simulink model "HvacCtrlrAirFlowDistbnFrnt"  (R2022b)
settings: Solver=FixedStepDiscrete  StartTime=0.0  StopTime=10.0  FixedStep=0.2  RelTol=1e-3
(part 2 of 2)

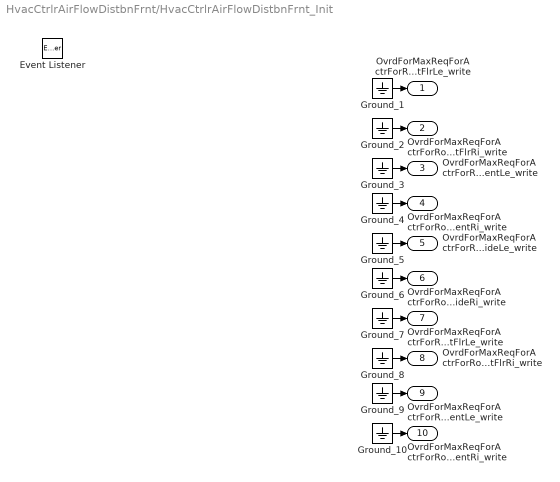
== system HvacCtrlrAirFlowDistbnFrnt/HvacCtrlrAirFlowDistbnFrnt_Init ==
  Event Listener : EventListener  EventName=reset
  Ground_{1..10} (10 alike) : Ground
  OvrdForMaxReqForActrForRowFirstAtFlr_OvrdForMaxReqForActrForRowFirstAtFlrLe_write : Outport
  OvrdForMaxReqForActrForRowFirstAtFlr_OvrdForMaxReqForActrForRowFirstAtFlrRi_write : Outport  Port=2
  OvrdForMaxReqForActrForRowFirstAtVent_OvrdForMaxReqForActrForRowFirstAtVentLe_write : Outport  Port=3
  OvrdForMaxReqForActrForRowFirstAtVent_OvrdForMaxReqForActrForRowFirstAtVentRi_write : Outport  Port=4
  OvrdForMaxReqForActrForRowFirstAtVent_OvrdForMaxReqForActrForRowFirstAtVentSideLe_write : Outport  Port=5
  OvrdForMaxReqForActrForRowFirstAtVent_OvrdForMaxReqForActrForRowFirstAtVentSideRi_write : Outport  Port=6
  OvrdForMaxReqForActrForRowSecAtFlr_OvrdForMaxReqForActrForRowSecAtFlrLe_write : Outport  Port=7
  OvrdForMaxReqForActrForRowSecAtFlr_OvrdForMaxReqForActrForRowSecAtFlrRi_write : Outport  Port=8
  OvrdForMaxReqForActrForRowSecAtVent_OvrdForMaxReqForActrForRowSecAtVentLe_write : Outport  Port=9
  OvrdForMaxReqForActrForRowSecAtVent_OvrdForMaxReqForActrForRowSecAtVentRi_write : Outport  Port=10
  wires:
  Ground_1/1 -> OvrdForMaxReqForActrForRowFirstAtFlr_OvrdForMaxReqForActrForRowFirstAtFlrLe_write/1
  Ground_2/1 -> OvrdForMaxReqForActrForRowFirstAtFlr_OvrdForMaxReqForActrForRowFirstAtFlrRi_write/1
  Ground_3/1 -> OvrdForMaxReqForActrForRowFirstAtVent_OvrdForMaxReqForActrForRowFirstAtVentLe_write/1
  Ground_4/1 -> OvrdForMaxReqForActrForRowFirstAtVent_OvrdForMaxReqForActrForRowFirstAtVentRi_write/1
  Ground_5/1 -> OvrdForMaxReqForActrForRowFirstAtVent_OvrdForMaxReqForActrForRowFirstAtVentSideLe_write/1
  Ground_6/1 -> OvrdForMaxReqForActrForRowFirstAtVent_OvrdForMaxReqForActrForRowFirstAtVentSideRi_write/1
  Ground_7/1 -> OvrdForMaxReqForActrForRowSecAtFlr_OvrdForMaxReqForActrForRowSecAtFlrLe_write/1
  Ground_8/1 -> OvrdForMaxReqForActrForRowSecAtFlr_OvrdForMaxReqForActrForRowSecAtFlrRi_write/1
  Ground_9/1 -> OvrdForMaxReqForActrForRowSecAtVent_OvrdForMaxReqForActrForRowSecAtVentLe_write/1
  Ground_10/1 -> OvrdForMaxReqForActrForRowSecAtVent_OvrdForMaxReqForActrForRowSecAtVentRi_write/1

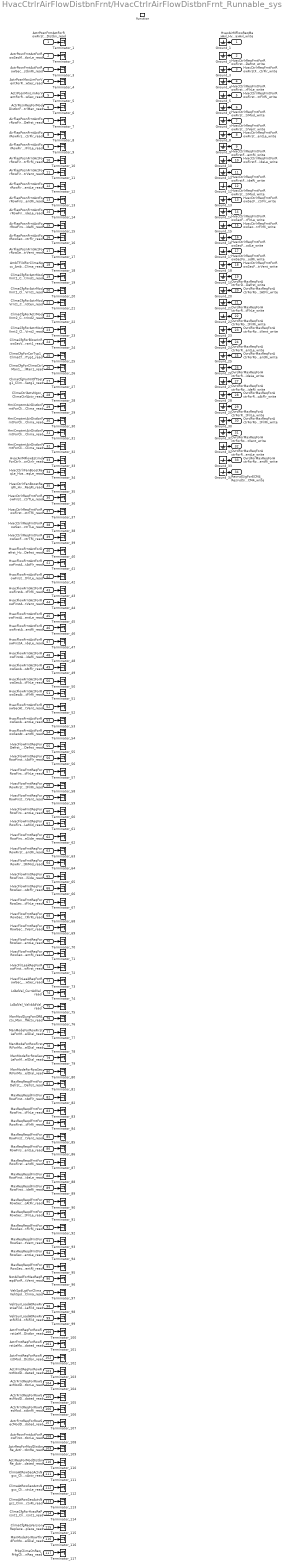
== system HvacCtrlrAirFlowDistbnFrnt/HvacCtrlrAirFlowDistbnFrnt_Runnable_sys ==
  ActrPosnFrntActForRowFirstModDistbn_ActrPosnFrntActForRowFirstModDistbn_read : Inport
  ActrPosnFrntActForRowSecModDistbnLe_ActrPosnFrntActForRowSecModDistbnLe_read : Inport  Port=2
  ActrPosnFrntActForRowSecModDistbnRi_ActrPosnFrntActForRowSecModDistbnRi_read : Inport  Port=3
  ActrPosnMaxLimForVentForRowSec_ActrPosnMaxLimForVentForRowSec_read : Inport  Port=4
  ActrPosnMinLimForVentForRowSec_ActrPosnMinLimForVentForRowSec_read : Inport  Port=5
  ActrPosnReqForModDistbnForClimaCtrlMan_ActrPosnReqForModDistbnForClimaCtrlMan_read : Inport  Port=6
  AirFlapPosnFrntActForRowFirstForActrDefrst_AirFlapPosnFrntActForRowFirstForActrDefrst_read : Inport  Port=7
  AirFlapPosnFrntActForRowFirstForActrFlr_AirFlapPosnFrntActForRowFirstForActrFlr_read : Inport  Port=8
  AirFlapPosnFrntActForRowFirstForActrFlrLe_AirFlapPosnFrntActForRowFirstForActrFlrLe_read : Inport  Port=9
  AirFlapPosnFrntActForRowFirstForActrFlrRi_AirFlapPosnFrntActForRowFirstForActrFlrRi_read : Inport  Port=10
  AirFlapPosnFrntActForRowFirstForActrVent_AirFlapPosnFrntActForRowFirstForActrVent_read : Inport  Port=11
  AirFlapPosnFrntActForRowFirstForActrVentLe_AirFlapPosnFrntActForRowFirstForActrVentLe_read : Inport  Port=12
  AirFlapPosnFrntActForRowFirstForActrVentRi_AirFlapPosnFrntActForRowFirstForActrVentRi_read : Inport  Port=13
  AirFlapPosnFrntActForRowFirstForActrVentSideLe_AirFlapPosnFrntActForRowFirstForActrVentSideLe_read : Inport  Port=14
  AirFlapPosnFrntActForRowFirstForActrVentSideRi_AirFlapPosnFrntActForRowFirstForActrVentSideRi_read : Inport  Port=15
  AirFlapPosnFrntActForRowSecForActrFlr_AirFlapPosnFrntActForRowSecForActrFlr_read : Inport  Port=16
  AirFlapPosnFrntActForRowSecForActrVent_AirFlapPosnFrntActForRowSecForActrVent_read : Inport  Port=17
  AmbTFildForClimaNgcc_AmbTFildForClima_read : Inport  Port=18
  ClimaCfgForActrModVrnt1_ClimaCfgForActrInd1_read : Inport  Port=19
  ClimaCfgForActrModVrnt1_ClimaCfgForActrModVrnt1_read : Inport  Port=20
  ClimaCfgForActrModVrnt1_ClimaCfgForActrModVrntCen_read : Inport  Port=21
  ClimaCfgForActrModVrnt2_ClimaCfgForActrInd2_read : Inport  Port=22
  ClimaCfgForActrModVrnt2_ClimaCfgForActrModVrnt2_read : Inport  Port=23
  ClimaCfgForBlowrInRowSecVentSidePrsnt1_ClimaCfgForBlowrInRowSecVentSidePrsnt1_read : Inport  Port=24
  ClimaCfgForCarTyp1_ClimaCfgForCarTyp1_read : Inport  Port=25
  ClimaCfgForClimaCtrlMan1_ClimaCfgForClimaCtrlMan1_read : Inport  Port=26
  ClimaCfgForNrOfTSetg1_ClimaCfgForNrOfTSetg1_read : Inport  Port=27
  ClimaCtrlActvNgcc_ClimaCtrlActv_read : Inport  Port=28
  HmiCmptmtAirDistbnFrntForClima_HmiCmptmtAirDistbnFrntForClima_read : Inport  Port=29
  HmiCmptmtAirDistbnFrntForClima_HmiCmptmtAirDistbnFrntRiForClima_read : Inport  Port=30
  HmiCmptmtAirDistbnFrntForClima_HmiCmptmtAirDistbnReForClima_read : Inport  Port=31
  HmiCmptmtAirDistbnFrntForClima_HmiCmptmtAirDistbnReRiForClima_read : Inport  Port=32
  HvacAirMFlowEstimdForCtrlr_HvacAirMFlowEstimdForCtrlr_read : Inport  Port=33
  HvacCtrlrFanBoostReqLe_HvacCtrlrFanBoostReqLe_read : Inport  Port=34
  HvacCtrlrFanBoostReqRi_HvacCtrlrFanBoostReqRi_read : Inport  Port=35
  HvacCtrlrReqFrntForRowFirstForActrTLe_HvacCtrlrReqFrntForRowFirstForActrTLe_read : Inport  Port=36
  HvacCtrlrReqFrntForRowFirstForActrTRi_HvacCtrlrReqFrntForRowFirstForActrTRi_read : Inport  Port=37
  HvacCtrlrReqFrntForRowSecForActrTLe_HvacCtrlrReqFrntForRowSecForActrTLe_read : Inport  Port=38
  HvacCtrlrReqFrntForRowSecForActrTRi_HvacCtrlrReqFrntForRowSecForActrTRi_read : Inport  Port=39
  HvacFlowFrntActForDefrst_HvacFlowFrntActForDefrst_read : Inport  Port=40
  HvacFlowFrntActForRowFirstAtFlr_HvacFlowFrntActForRowFirstAtFlr_read : Inport  Port=41
  HvacFlowFrntActForRowFirstAtFlr_HvacFlowFrntActForRowFirstAtFlrLe_read : Inport  Port=42
  HvacFlowFrntActForRowFirstAtFlr_HvacFlowFrntActForRowFirstAtFlrRi_read : Inport  Port=43
  HvacFlowFrntActForRowFirstAtVent_HvacFlowFrntActForRowFirstAtVent_read : Inport  Port=44
  HvacFlowFrntActForRowFirstAtVent_HvacFlowFrntActForRowFirstAtVentLe_read : Inport  Port=45
  HvacFlowFrntActForRowFirstAtVent_HvacFlowFrntActForRowFirstAtVentRi_read : Inport  Port=46
  HvacFlowFrntActForRowFirstAtVent_HvacFlowFrntActForRowFirstAtVentSideLe_read : Inport  Port=47
  HvacFlowFrntActForRowFirstAtVent_HvacFlowFrntActForRowFirstAtVentSideRi_read : Inport  Port=48
  HvacFlowFrntActForRowSecAtFlr_HvacFlowFrntActForRowSecAtFlr_read : Inport  Port=49
  HvacFlowFrntActForRowSecAtFlr_HvacFlowFrntActForRowSecAtFlrLe_read : Inport  Port=50
  HvacFlowFrntActForRowSecAtFlr_HvacFlowFrntActForRowSecAtFlrRi_read : Inport  Port=51
  HvacFlowFrntActForRowSecAtVent_HvacFlowFrntActForRowSecAtVent_read : Inport  Port=52
  HvacFlowFrntActForRowSecAtVent_HvacFlowFrntActForRowSecAtVentLe_read : Inport  Port=53
  HvacFlowFrntActForRowSecAtVent_HvacFlowFrntActForRowSecAtVentRi_read : Inport  Port=54
  HvacFlowFrntReqForDefrst_HvacFlowFrntReqForDefrst_read : Inport  Port=55
  HvacFlowFrntReqForRowFirstAtFlr_HvacFlowFrntReqForRowFirstAtFlr_read : Inport  Port=56
  HvacFlowFrntReqForRowFirstAtFlr_HvacFlowFrntReqForRowFirstAtFlrLe_read : Inport  Port=57
  HvacFlowFrntReqForRowFirstAtFlr_HvacFlowFrntReqForRowFirstAtFlrRi_read : Inport  Port=58
  HvacFlowFrntReqForRowFirstAtVent_HvacFlowFrntReqForRowFirstAtVent_read : Inport  Port=59
  HvacFlowFrntReqForRowFirstAtVent_HvacFlowFrntReqForRowFirstAtVentLe_read : Inport  Port=60
  HvacFlowFrntReqForRowFirstAtVent_HvacFlowFrntReqForRowFirstAtVentLeMid_read : Inport  Port=61
  HvacFlowFrntReqForRowFirstAtVent_HvacFlowFrntReqForRowFirstAtVentLeSide_read : Inport  Port=62
  HvacFlowFrntReqForRowFirstAtVent_HvacFlowFrntReqForRowFirstAtVentRi_read : Inport  Port=63
  HvacFlowFrntReqForRowFirstAtVent_HvacFlowFrntReqForRowFirstAtVentRiMid_read : Inport  Port=64
  HvacFlowFrntReqForRowFirstAtVent_HvacFlowFrntReqForRowFirstAtVentRiSide_read : Inport  Port=65
  HvacFlowFrntReqForRowSecAtFlr_HvacFlowFrntReqForRowSecAtFlr_read : Inport  Port=66
  HvacFlowFrntReqForRowSecAtFlr_HvacFlowFrntReqForRowSecAtFlrLe_read : Inport  Port=67
  HvacFlowFrntReqForRowSecAtFlr_HvacFlowFrntReqForRowSecAtFlrRi_read : Inport  Port=68
  HvacFlowFrntReqForRowSecAtVent_HvacFlowFrntReqForRowSecAtVent_read : Inport  Port=69
  HvacFlowFrntReqForRowSecAtVent_HvacFlowFrntReqForRowSecAtVentLe_read : Inport  Port=70
  HvacFlowFrntReqForRowSecAtVent_HvacFlowFrntReqForRowSecAtVentRi_read : Inport  Port=71
  HvacFlrLeakReqForRowFirst_HvacFlrLeakReqForRowFirst_read : Inport  Port=72
  HvacFlrLeakReqForRowSec_HvacFlrLeakReqForRowSec_read : Inport  Port=73
  LdAdVal_CurrAdVal_read : Inport  Port=74
  LdAdVal_ValidAdVal_read : Inport  Port=75
  ManModDurgFanOffActv_ManModDurgFanOffActv_read : Inport  Port=76
  ManModeForRowFirstLeForModelDial_ManModeForRowFirstLeForModelDial_read : Inport  Port=77
  ManModeForRowFirstRiForModelDial_ManModeForRowFirstRiForModelDial_read : Inport  Port=78
  ManModeForRowSecLeForModelDial_ManModeForRowSecLeForModelDial_read : Inport  Port=79
  ManModeForRowSecRiForModelDial_ManModeForRowSecRiForModelDial_read : Inport  Port=80
  MaxReqReqdFrntForDefrst_MaxReqReqdFrntForDefrst_read : Inport  Port=81
  MaxReqReqdFrntForRowFirstAtFlr_MaxReqReqdFrntForRowFirstAtFlr_read : Inport  Port=82
  MaxReqReqdFrntForRowFirstAtFlr_MaxReqReqdFrntForRowFirstAtFlrLe_read : Inport  Port=83
  MaxReqReqdFrntForRowFirstAtFlr_MaxReqReqdFrntForRowFirstAtFlrRi_read : Inport  Port=84
  MaxReqReqdFrntForRowFirstAtVent_MaxReqReqdFrntForRowFirstAtVent_read : Inport  Port=85
  MaxReqReqdFrntForRowFirstAtVent_MaxReqReqdFrntForRowFirstAtVentLe_read : Inport  Port=86
  MaxReqReqdFrntForRowFirstAtVent_MaxReqReqdFrntForRowFirstAtVentRi_read : Inport  Port=87
  MaxReqReqdFrntForRowFirstAtVent_MaxReqReqdFrntForRowFirstAtVentSideLe_read : Inport  Port=88
  MaxReqReqdFrntForRowFirstAtVent_MaxReqReqdFrntForRowFirstAtVentSideRi_read : Inport  Port=89
  MaxReqReqdFrntForRowSecAtFlr_MaxReqReqdFrntForRowSecAtFlr_read : Inport  Port=90
  MaxReqReqdFrntForRowSecAtFlr_MaxReqReqdFrntForRowSecAtFlrLe_read : Inport  Port=91
  MaxReqReqdFrntForRowSecAtFlr_MaxReqReqdFrntForRowSecAtFlrRi_read : Inport  Port=92
  MaxReqReqdFrntForRowSecAtVent_MaxReqReqdFrntForRowSecAtVent_read : Inport  Port=93
  MaxReqReqdFrntForRowSecAtVent_MaxReqReqdFrntForRowSecAtVentLe_read : Inport  Port=94
  MaxReqReqdFrntForRowSecAtVent_MaxReqReqdFrntForRowSecAtVentRi_read : Inport  Port=95
  NotAllwdForMaxReqReqdForRowSecAtVent_NotAllwdForMaxReqReqdForRowSecAtVent_read : Inport  Port=96
  VehSpdLgtForClima_VehSpdLgtForClima_read : Inport  Port=97
  VehSunLoadAtRowFirstLeFild_VehSunLoadAtRowFirstLeFild_read : Inport  Port=98
  VehSunLoadAtRowFirstRiFild_VehSunLoadAtRowFirstRiFild_read : Inport  Port=99
  ActrFrntReqForRowFirstLeModDistbn_ActrFrntReqForRowFirstLeModDistbn_read : Inport  Port=100
  ActrFrntReqForRowFirstLeModDistbn_ActrFrntReqForRowFirstLeModDistbn_IsUpdated_read : Inport  Port=101
  ActrFrntReqForRowFirstModDistbn_ActrFrntReqForRowFirstModDistbn_read : Inport  Port=102
  ActrFrntReqForRowFirstModDistbn_ActrFrntReqForRowFirstModDistbn_IsUpdated_read : Inport  Port=103
  ActrFrntReqForRowSecModDistbnLe_ActrFrntReqForRowSecModDistbnLe_read : Inport  Port=104
  ActrFrntReqForRowSecModDistbnLe_ActrFrntReqForRowSecModDistbnLe_IsUpdated_read : Inport  Port=105
  ActrFrntReqForRowSecModDistbnRi_ActrFrntReqForRowSecModDistbnRi_read : Inport  Port=106
  ActrFrntReqForRowSecModDistbnRi_ActrFrntReqForRowSecModDistbnRi_IsUpdated_read : Inport  Port=107
  ActrPosnFrntActForRowFirstModDistbnLe_ActrPosnFrntActForRowFirstModDistbnLe_read : Inport  Port=108
  ActrReqForModDistbnRe_ActrReqForModDistbnRe_read : Inport  Port=109
  ActrReqForModDistbnRe_ActrReqForModDistbnRe_IsUpdated_read : Inport  Port=110
  ClimaAtRowSecActvNgcc_ClimaAtRowSecActv_read : Inport  Port=111
  ClimaAtRowSecActvNgcc_ClimaAtRowSecActvLe_read : Inport  Port=112
  ClimaAtRowSecActvNgcc_ClimaAtRowSecActvRi_read : Inport  Port=113
  ClimaCfgForHvacRePrsnt1_ClimaCfgForHvacRePrsnt1_read : Inport  Port=114
  ClimaCfgReqVersionReplace_ClimaCfgReqVersionReplace_read : Inport  Port=115
  ManModeForRowThrdForModelDial_ManModeForRowThrdForModelDial_read : Inport  Port=116
  PrkgClimaOnReq_PrkgClimaOnReq_read : Inport  Port=117
  function : TriggerPort  TriggerType=function-call  VariantControl=(inherit)  SampleTimeType=periodic  SampleTime=-1
  Ground_{1..34} (34 alike) : Ground
  Terminator_{1..117} (117 alike) : Terminator
  HvacAirMFlowReqMaxAvl_HvacAirMFlowReqMaxAvl_write : Outport
      settings: EnsureOutportIsVirtual=on
  HvacCtrlrReqFrntForRowFirstForActrDefrst_HvacCtrlrReqFrntForRowFirstForActrDefrst_write : Outport  Port=2
      settings: EnsureOutportIsVirtual=on
  HvacCtrlrReqFrntForRowFirstForActrFlr_HvacCtrlrReqFrntForRowFirstForActrFlr_write : Outport  Port=3
      settings: EnsureOutportIsVirtual=on
  HvacCtrlrReqFrntForRowFirstForActrFlr_HvacCtrlrReqFrntForRowFirstForActrFlrLe_write : Outport  Port=4
      settings: EnsureOutportIsVirtual=on
  HvacCtrlrReqFrntForRowFirstForActrFlr_HvacCtrlrReqFrntForRowFirstForActrFlrRi_write : Outport  Port=5
      settings: EnsureOutportIsVirtual=on
  HvacCtrlrReqFrntForRowFirstForActrMod_HvacCtrlrReqFrntForRowFirstForActrMod_write : Outport  Port=6
      settings: EnsureOutportIsVirtual=on
  HvacCtrlrReqFrntForRowFirstForActrVent_HvacCtrlrReqFrntForRowFirstForActrVent_write : Outport  Port=7
      settings: EnsureOutportIsVirtual=on
  HvacCtrlrReqFrntForRowFirstForActrVent_HvacCtrlrReqFrntForRowFirstForActrVentLe_write : Outport  Port=8
      settings: EnsureOutportIsVirtual=on
  HvacCtrlrReqFrntForRowFirstForActrVent_HvacCtrlrReqFrntForRowFirstForActrVentRi_write : Outport  Port=9
      settings: EnsureOutportIsVirtual=on
  HvacCtrlrReqFrntForRowFirstForActrVent_HvacCtrlrReqFrntForRowFirstForActrVentSideLe_write : Outport  Port=10
      settings: EnsureOutportIsVirtual=on
  HvacCtrlrReqFrntForRowFirstForActrVent_HvacCtrlrReqFrntForRowFirstForActrVentSideRi_write : Outport  Port=11
      settings: EnsureOutportIsVirtual=on
  HvacCtrlrReqFrntForRowFirstLeForActrMod_HvacCtrlrReqFrntForRowFirstLeForActrMod_write : Outport  Port=12
      settings: EnsureOutportIsVirtual=on
  HvacCtrlrReqFrntForRowSecForActrFlr_HvacCtrlrReqFrntForRowSecForActrFlr_write : Outport  Port=13
      settings: EnsureOutportIsVirtual=on
  HvacCtrlrReqFrntForRowSecForActrFlr_HvacCtrlrReqFrntForRowSecForActrFlrLe_write : Outport  Port=14
      settings: EnsureOutportIsVirtual=on
  HvacCtrlrReqFrntForRowSecForActrFlr_HvacCtrlrReqFrntForRowSecForActrFlrRi_write : Outport  Port=15
      settings: EnsureOutportIsVirtual=on
  HvacCtrlrReqFrntForRowSecForActrModLe_HvacCtrlrReqFrntForRowSecForActrModLe_write : Outport  Port=16
      settings: EnsureOutportIsVirtual=on
  HvacCtrlrReqFrntForRowSecForActrModRi_HvacCtrlrReqFrntForRowSecForActrModRi_write : Outport  Port=17
      settings: EnsureOutportIsVirtual=on
  HvacCtrlrReqFrntForRowSecForActrVent_HvacCtrlrReqFrntForRowSecForActrVent_write : Outport  Port=18
      settings: EnsureOutportIsVirtual=on
  OvrdForMaxReqForActrForDefrst_OvrdForMaxReqForActrForDefrst_write : Outport  Port=19
      settings: EnsureOutportIsVirtual=on
  OvrdForMaxReqForActrForRowFirstAtFlr_OvrdForMaxReqForActrForRowFirstAtFlr_write : Outport  Port=20
      settings: EnsureOutportIsVirtual=on
  OvrdForMaxReqForActrForRowFirstAtFlr_OvrdForMaxReqForActrForRowFirstAtFlrLe_write : Outport  Port=21
      settings: EnsureOutportIsVirtual=on
  OvrdForMaxReqForActrForRowFirstAtFlr_OvrdForMaxReqForActrForRowFirstAtFlrRi_write : Outport  Port=22
      settings: EnsureOutportIsVirtual=on
  OvrdForMaxReqForActrForRowFirstAtVent_OvrdForMaxReqForActrForRowFirstAtVent_write : Outport  Port=23
      settings: EnsureOutportIsVirtual=on
  OvrdForMaxReqForActrForRowFirstAtVent_OvrdForMaxReqForActrForRowFirstAtVentLe_write : Outport  Port=24
      settings: EnsureOutportIsVirtual=on
  OvrdForMaxReqForActrForRowFirstAtVent_OvrdForMaxReqForActrForRowFirstAtVentRi_write : Outport  Port=25
      settings: EnsureOutportIsVirtual=on
  OvrdForMaxReqForActrForRowFirstAtVent_OvrdForMaxReqForActrForRowFirstAtVentSideLe_write : Outport  Port=26
      settings: EnsureOutportIsVirtual=on
  OvrdForMaxReqForActrForRowFirstAtVent_OvrdForMaxReqForActrForRowFirstAtVentSideRi_write : Outport  Port=27
      settings: EnsureOutportIsVirtual=on
  OvrdForMaxReqForActrForRowSecAtFlr_OvrdForMaxReqForActrForRowSecAtFlr_write : Outport  Port=28
      settings: EnsureOutportIsVirtual=on
  OvrdForMaxReqForActrForRowSecAtFlr_OvrdForMaxReqForActrForRowSecAtFlrLe_write : Outport  Port=29
      settings: EnsureOutportIsVirtual=on
  OvrdForMaxReqForActrForRowSecAtFlr_OvrdForMaxReqForActrForRowSecAtFlrRi_write : Outport  Port=30
      settings: EnsureOutportIsVirtual=on
  OvrdForMaxReqForActrForRowSecAtVent_OvrdForMaxReqForActrForRowSecAtVent_write : Outport  Port=31
      settings: EnsureOutportIsVirtual=on
  OvrdForMaxReqForActrForRowSecAtVent_OvrdForMaxReqForActrForRowSecAtVentLe_write : Outport  Port=32
      settings: EnsureOutportIsVirtual=on
  OvrdForMaxReqForActrForRowSecAtVent_OvrdForMaxReqForActrForRowSecAtVentRi_write : Outport  Port=33
      settings: EnsureOutportIsVirtual=on
  ResrvdSigForECM4_ResrvdSigForECM4_write : Outport  Port=34
      settings: EnsureOutportIsVirtual=on
  wires:
  ActrPosnFrntActForRowFirstModDistbn_ActrPosnFrntActForRowFirstModDistbn_read/1 -> Terminator_1/1
  ActrPosnFrntActForRowSecModDistbnLe_ActrPosnFrntActForRowSecModDistbnLe_read/1 -> Terminator_2/1
  ActrPosnFrntActForRowSecModDistbnRi_ActrPosnFrntActForRowSecModDistbnRi_read/1 -> Terminator_3/1
  ActrPosnMaxLimForVentForRowSec_ActrPosnMaxLimForVentForRowSec_read/1 -> Terminator_4/1
  ActrPosnMinLimForVentForRowSec_ActrPosnMinLimForVentForRowSec_read/1 -> Terminator_5/1
  ActrPosnReqForModDistbnForClimaCtrlMan_ActrPosnReqForModDistbnForClimaCtrlMan_read/1 -> Terminator_6/1
  AirFlapPosnFrntActForRowFirstForActrDefrst_AirFlapPosnFrntActForRowFirstForActrDefrst_read/1 -> Terminator_7/1
  AirFlapPosnFrntActForRowFirstForActrFlr_AirFlapPosnFrntActForRowFirstForActrFlr_read/1 -> Terminator_8/1
  AirFlapPosnFrntActForRowFirstForActrFlrLe_AirFlapPosnFrntActForRowFirstForActrFlrLe_read/1 -> Terminator_9/1
  AirFlapPosnFrntActForRowFirstForActrFlrRi_AirFlapPosnFrntActForRowFirstForActrFlrRi_read/1 -> Terminator_10/1
  AirFlapPosnFrntActForRowFirstForActrVent_AirFlapPosnFrntActForRowFirstForActrVent_read/1 -> Terminator_11/1
  AirFlapPosnFrntActForRowFirstForActrVentLe_AirFlapPosnFrntActForRowFirstForActrVentLe_read/1 -> Terminator_12/1
  AirFlapPosnFrntActForRowFirstForActrVentRi_AirFlapPosnFrntActForRowFirstForActrVentRi_read/1 -> Terminator_13/1
  AirFlapPosnFrntActForRowFirstForActrVentSideLe_AirFlapPosnFrntActForRowFirstForActrVentSideLe_read/1 -> Terminator_14/1
  AirFlapPosnFrntActForRowFirstForActrVentSideRi_AirFlapPosnFrntActForRowFirstForActrVentSideRi_read/1 -> Terminator_15/1
  AirFlapPosnFrntActForRowSecForActrFlr_AirFlapPosnFrntActForRowSecForActrFlr_read/1 -> Terminator_16/1
  AirFlapPosnFrntActForRowSecForActrVent_AirFlapPosnFrntActForRowSecForActrVent_read/1 -> Terminator_17/1
  AmbTFildForClimaNgcc_AmbTFildForClima_read/1 -> Terminator_18/1
  ClimaCfgForActrModVrnt1_ClimaCfgForActrInd1_read/1 -> Terminator_19/1
  ClimaCfgForActrModVrnt1_ClimaCfgForActrModVrnt1_read/1 -> Terminator_20/1
  ClimaCfgForActrModVrnt1_ClimaCfgForActrModVrntCen_read/1 -> Terminator_21/1
  ClimaCfgForActrModVrnt2_ClimaCfgForActrInd2_read/1 -> Terminator_22/1
  ClimaCfgForActrModVrnt2_ClimaCfgForActrModVrnt2_read/1 -> Terminator_23/1
  ClimaCfgForBlowrInRowSecVentSidePrsnt1_ClimaCfgForBlowrInRowSecVentSidePrsnt1_read/1 -> Terminator_24/1
  ClimaCfgForCarTyp1_ClimaCfgForCarTyp1_read/1 -> Terminator_25/1
  ClimaCfgForClimaCtrlMan1_ClimaCfgForClimaCtrlMan1_read/1 -> Terminator_26/1
  ClimaCfgForNrOfTSetg1_ClimaCfgForNrOfTSetg1_read/1 -> Terminator_27/1
  ClimaCtrlActvNgcc_ClimaCtrlActv_read/1 -> Terminator_28/1
  HmiCmptmtAirDistbnFrntForClima_HmiCmptmtAirDistbnFrntForClima_read/1 -> Terminator_29/1
  HmiCmptmtAirDistbnFrntForClima_HmiCmptmtAirDistbnFrntRiForClima_read/1 -> Terminator_30/1
  HmiCmptmtAirDistbnFrntForClima_HmiCmptmtAirDistbnReForClima_read/1 -> Terminator_31/1
  HmiCmptmtAirDistbnFrntForClima_HmiCmptmtAirDistbnReRiForClima_read/1 -> Terminator_32/1
  HvacAirMFlowEstimdForCtrlr_HvacAirMFlowEstimdForCtrlr_read/1 -> Terminator_33/1
  HvacCtrlrFanBoostReqLe_HvacCtrlrFanBoostReqLe_read/1 -> Terminator_34/1
  HvacCtrlrFanBoostReqRi_HvacCtrlrFanBoostReqRi_read/1 -> Terminator_35/1
  HvacCtrlrReqFrntForRowFirstForActrTLe_HvacCtrlrReqFrntForRowFirstForActrTLe_read/1 -> Terminator_36/1
  HvacCtrlrReqFrntForRowFirstForActrTRi_HvacCtrlrReqFrntForRowFirstForActrTRi_read/1 -> Terminator_37/1
  HvacCtrlrReqFrntForRowSecForActrTLe_HvacCtrlrReqFrntForRowSecForActrTLe_read/1 -> Terminator_38/1
  HvacCtrlrReqFrntForRowSecForActrTRi_HvacCtrlrReqFrntForRowSecForActrTRi_read/1 -> Terminator_39/1
  HvacFlowFrntActForDefrst_HvacFlowFrntActForDefrst_read/1 -> Terminator_40/1
  HvacFlowFrntActForRowFirstAtFlr_HvacFlowFrntActForRowFirstAtFlr_read/1 -> Terminator_41/1
  HvacFlowFrntActForRowFirstAtFlr_HvacFlowFrntActForRowFirstAtFlrLe_read/1 -> Terminator_42/1
  HvacFlowFrntActForRowFirstAtFlr_HvacFlowFrntActForRowFirstAtFlrRi_read/1 -> Terminator_43/1
  HvacFlowFrntActForRowFirstAtVent_HvacFlowFrntActForRowFirstAtVent_read/1 -> Terminator_44/1
  HvacFlowFrntActForRowFirstAtVent_HvacFlowFrntActForRowFirstAtVentLe_read/1 -> Terminator_45/1
  HvacFlowFrntActForRowFirstAtVent_HvacFlowFrntActForRowFirstAtVentRi_read/1 -> Terminator_46/1
  HvacFlowFrntActForRowFirstAtVent_HvacFlowFrntActForRowFirstAtVentSideLe_read/1 -> Terminator_47/1
  HvacFlowFrntActForRowFirstAtVent_HvacFlowFrntActForRowFirstAtVentSideRi_read/1 -> Terminator_48/1
  HvacFlowFrntActForRowSecAtFlr_HvacFlowFrntActForRowSecAtFlr_read/1 -> Terminator_49/1
  HvacFlowFrntActForRowSecAtFlr_HvacFlowFrntActForRowSecAtFlrLe_read/1 -> Terminator_50/1
  HvacFlowFrntActForRowSecAtFlr_HvacFlowFrntActForRowSecAtFlrRi_read/1 -> Terminator_51/1
  HvacFlowFrntActForRowSecAtVent_HvacFlowFrntActForRowSecAtVent_read/1 -> Terminator_52/1
  HvacFlowFrntActForRowSecAtVent_HvacFlowFrntActForRowSecAtVentLe_read/1 -> Terminator_53/1
  HvacFlowFrntActForRowSecAtVent_HvacFlowFrntActForRowSecAtVentRi_read/1 -> Terminator_54/1
  HvacFlowFrntReqForDefrst_HvacFlowFrntReqForDefrst_read/1 -> Terminator_55/1
  HvacFlowFrntReqForRowFirstAtFlr_HvacFlowFrntReqForRowFirstAtFlr_read/1 -> Terminator_56/1
  HvacFlowFrntReqForRowFirstAtFlr_HvacFlowFrntReqForRowFirstAtFlrLe_read/1 -> Terminator_57/1
  HvacFlowFrntReqForRowFirstAtFlr_HvacFlowFrntReqForRowFirstAtFlrRi_read/1 -> Terminator_58/1
  HvacFlowFrntReqForRowFirstAtVent_HvacFlowFrntReqForRowFirstAtVent_read/1 -> Terminator_59/1
  HvacFlowFrntReqForRowFirstAtVent_HvacFlowFrntReqForRowFirstAtVentLe_read/1 -> Terminator_60/1
  HvacFlowFrntReqForRowFirstAtVent_HvacFlowFrntReqForRowFirstAtVentLeMid_read/1 -> Terminator_61/1
  HvacFlowFrntReqForRowFirstAtVent_HvacFlowFrntReqForRowFirstAtVentLeSide_read/1 -> Terminator_62/1
  HvacFlowFrntReqForRowFirstAtVent_HvacFlowFrntReqForRowFirstAtVentRi_read/1 -> Terminator_63/1
  HvacFlowFrntReqForRowFirstAtVent_HvacFlowFrntReqForRowFirstAtVentRiMid_read/1 -> Terminator_64/1
  HvacFlowFrntReqForRowFirstAtVent_HvacFlowFrntReqForRowFirstAtVentRiSide_read/1 -> Terminator_65/1
  HvacFlowFrntReqForRowSecAtFlr_HvacFlowFrntReqForRowSecAtFlr_read/1 -> Terminator_66/1
  HvacFlowFrntReqForRowSecAtFlr_HvacFlowFrntReqForRowSecAtFlrLe_read/1 -> Terminator_67/1
  HvacFlowFrntReqForRowSecAtFlr_HvacFlowFrntReqForRowSecAtFlrRi_read/1 -> Terminator_68/1
  HvacFlowFrntReqForRowSecAtVent_HvacFlowFrntReqForRowSecAtVent_read/1 -> Terminator_69/1
  HvacFlowFrntReqForRowSecAtVent_HvacFlowFrntReqForRowSecAtVentLe_read/1 -> Terminator_70/1
  HvacFlowFrntReqForRowSecAtVent_HvacFlowFrntReqForRowSecAtVentRi_read/1 -> Terminator_71/1
  HvacFlrLeakReqForRowFirst_HvacFlrLeakReqForRowFirst_read/1 -> Terminator_72/1
  HvacFlrLeakReqForRowSec_HvacFlrLeakReqForRowSec_read/1 -> Terminator_73/1
  LdAdVal_CurrAdVal_read/1 -> Terminator_74/1
  LdAdVal_ValidAdVal_read/1 -> Terminator_75/1
  ManModDurgFanOffActv_ManModDurgFanOffActv_read/1 -> Terminator_76/1
  ManModeForRowFirstLeForModelDial_ManModeForRowFirstLeForModelDial_read/1 -> Terminator_77/1
  ManModeForRowFirstRiForModelDial_ManModeForRowFirstRiForModelDial_read/1 -> Terminator_78/1
  ManModeForRowSecLeForModelDial_ManModeForRowSecLeForModelDial_read/1 -> Terminator_79/1
  ManModeForRowSecRiForModelDial_ManModeForRowSecRiForModelDial_read/1 -> Terminator_80/1
  MaxReqReqdFrntForDefrst_MaxReqReqdFrntForDefrst_read/1 -> Terminator_81/1
  MaxReqReqdFrntForRowFirstAtFlr_MaxReqReqdFrntForRowFirstAtFlr_read/1 -> Terminator_82/1
  MaxReqReqdFrntForRowFirstAtFlr_MaxReqReqdFrntForRowFirstAtFlrLe_read/1 -> Terminator_83/1
  MaxReqReqdFrntForRowFirstAtFlr_MaxReqReqdFrntForRowFirstAtFlrRi_read/1 -> Terminator_84/1
  MaxReqReqdFrntForRowFirstAtVent_MaxReqReqdFrntForRowFirstAtVent_read/1 -> Terminator_85/1
  MaxReqReqdFrntForRowFirstAtVent_MaxReqReqdFrntForRowFirstAtVentLe_read/1 -> Terminator_86/1
  MaxReqReqdFrntForRowFirstAtVent_MaxReqReqdFrntForRowFirstAtVentRi_read/1 -> Terminator_87/1
  MaxReqReqdFrntForRowFirstAtVent_MaxReqReqdFrntForRowFirstAtVentSideLe_read/1 -> Terminator_88/1
  MaxReqReqdFrntForRowFirstAtVent_MaxReqReqdFrntForRowFirstAtVentSideRi_read/1 -> Terminator_89/1
  MaxReqReqdFrntForRowSecAtFlr_MaxReqReqdFrntForRowSecAtFlr_read/1 -> Terminator_90/1
  MaxReqReqdFrntForRowSecAtFlr_MaxReqReqdFrntForRowSecAtFlrLe_read/1 -> Terminator_91/1
  MaxReqReqdFrntForRowSecAtFlr_MaxReqReqdFrntForRowSecAtFlrRi_read/1 -> Terminator_92/1
  MaxReqReqdFrntForRowSecAtVent_MaxReqReqdFrntForRowSecAtVent_read/1 -> Terminator_93/1
  MaxReqReqdFrntForRowSecAtVent_MaxReqReqdFrntForRowSecAtVentLe_read/1 -> Terminator_94/1
  MaxReqReqdFrntForRowSecAtVent_MaxReqReqdFrntForRowSecAtVentRi_read/1 -> Terminator_95/1
  NotAllwdForMaxReqReqdForRowSecAtVent_NotAllwdForMaxReqReqdForRowSecAtVent_read/1 -> Terminator_96/1
  VehSpdLgtForClima_VehSpdLgtForClima_read/1 -> Terminator_97/1
  VehSunLoadAtRowFirstLeFild_VehSunLoadAtRowFirstLeFild_read/1 -> Terminator_98/1
  VehSunLoadAtRowFirstRiFild_VehSunLoadAtRowFirstRiFild_read/1 -> Terminator_99/1
  ActrFrntReqForRowFirstLeModDistbn_ActrFrntReqForRowFirstLeModDistbn_read/1 -> Terminator_100/1
  ActrFrntReqForRowFirstLeModDistbn_ActrFrntReqForRowFirstLeModDistbn_IsUpdated_read/1 -> Terminator_101/1
  ActrFrntReqForRowFirstModDistbn_ActrFrntReqForRowFirstModDistbn_read/1 -> Terminator_102/1
  ActrFrntReqForRowFirstModDistbn_ActrFrntReqForRowFirstModDistbn_IsUpdated_read/1 -> Terminator_103/1
  ActrFrntReqForRowSecModDistbnLe_ActrFrntReqForRowSecModDistbnLe_read/1 -> Terminator_104/1
  ActrFrntReqForRowSecModDistbnLe_ActrFrntReqForRowSecModDistbnLe_IsUpdated_read/1 -> Terminator_105/1
  ActrFrntReqForRowSecModDistbnRi_ActrFrntReqForRowSecModDistbnRi_read/1 -> Terminator_106/1
  ActrFrntReqForRowSecModDistbnRi_ActrFrntReqForRowSecModDistbnRi_IsUpdated_read/1 -> Terminator_107/1
  ActrPosnFrntActForRowFirstModDistbnLe_ActrPosnFrntActForRowFirstModDistbnLe_read/1 -> Terminator_108/1
  ActrReqForModDistbnRe_ActrReqForModDistbnRe_read/1 -> Terminator_109/1
  ActrReqForModDistbnRe_ActrReqForModDistbnRe_IsUpdated_read/1 -> Terminator_110/1
  ClimaAtRowSecActvNgcc_ClimaAtRowSecActv_read/1 -> Terminator_111/1
  ClimaAtRowSecActvNgcc_ClimaAtRowSecActvLe_read/1 -> Terminator_112/1
  ClimaAtRowSecActvNgcc_ClimaAtRowSecActvRi_read/1 -> Terminator_113/1
  ClimaCfgForHvacRePrsnt1_ClimaCfgForHvacRePrsnt1_read/1 -> Terminator_114/1
  ClimaCfgReqVersionReplace_ClimaCfgReqVersionReplace_read/1 -> Terminator_115/1
  ManModeForRowThrdForModelDial_ManModeForRowThrdForModelDial_read/1 -> Terminator_116/1
  PrkgClimaOnReq_PrkgClimaOnReq_read/1 -> Terminator_117/1
  Ground_1/1 -> HvacAirMFlowReqMaxAvl_HvacAirMFlowReqMaxAvl_write/1
  Ground_2/1 -> HvacCtrlrReqFrntForRowFirstForActrDefrst_HvacCtrlrReqFrntForRowFirstForActrDefrst_write/1
  Ground_3/1 -> HvacCtrlrReqFrntForRowFirstForActrFlr_HvacCtrlrReqFrntForRowFirstForActrFlr_write/1
  Ground_4/1 -> HvacCtrlrReqFrntForRowFirstForActrFlr_HvacCtrlrReqFrntForRowFirstForActrFlrLe_write/1
  Ground_5/1 -> HvacCtrlrReqFrntForRowFirstForActrFlr_HvacCtrlrReqFrntForRowFirstForActrFlrRi_write/1
  Ground_6/1 -> HvacCtrlrReqFrntForRowFirstForActrMod_HvacCtrlrReqFrntForRowFirstForActrMod_write/1
  Ground_7/1 -> HvacCtrlrReqFrntForRowFirstForActrVent_HvacCtrlrReqFrntForRowFirstForActrVent_write/1
  Ground_8/1 -> HvacCtrlrReqFrntForRowFirstForActrVent_HvacCtrlrReqFrntForRowFirstForActrVentLe_write/1
  Ground_9/1 -> HvacCtrlrReqFrntForRowFirstForActrVent_HvacCtrlrReqFrntForRowFirstForActrVentRi_write/1
  Ground_10/1 -> HvacCtrlrReqFrntForRowFirstForActrVent_HvacCtrlrReqFrntForRowFirstForActrVentSideLe_write/1
  Ground_11/1 -> HvacCtrlrReqFrntForRowFirstForActrVent_HvacCtrlrReqFrntForRowFirstForActrVentSideRi_write/1
  Ground_12/1 -> HvacCtrlrReqFrntForRowFirstLeForActrMod_HvacCtrlrReqFrntForRowFirstLeForActrMod_write/1
  Ground_13/1 -> HvacCtrlrReqFrntForRowSecForActrFlr_HvacCtrlrReqFrntForRowSecForActrFlr_write/1
  Ground_14/1 -> HvacCtrlrReqFrntForRowSecForActrFlr_HvacCtrlrReqFrntForRowSecForActrFlrLe_write/1
  Ground_15/1 -> HvacCtrlrReqFrntForRowSecForActrFlr_HvacCtrlrReqFrntForRowSecForActrFlrRi_write/1
  Ground_16/1 -> HvacCtrlrReqFrntForRowSecForActrModLe_HvacCtrlrReqFrntForRowSecForActrModLe_write/1
  Ground_17/1 -> HvacCtrlrReqFrntForRowSecForActrModRi_HvacCtrlrReqFrntForRowSecForActrModRi_write/1
  Ground_18/1 -> HvacCtrlrReqFrntForRowSecForActrVent_HvacCtrlrReqFrntForRowSecForActrVent_write/1
  Ground_19/1 -> OvrdForMaxReqForActrForDefrst_OvrdForMaxReqForActrForDefrst_write/1
  Ground_20/1 -> OvrdForMaxReqForActrForRowFirstAtFlr_OvrdForMaxReqForActrForRowFirstAtFlr_write/1
  Ground_21/1 -> OvrdForMaxReqForActrForRowFirstAtFlr_OvrdForMaxReqForActrForRowFirstAtFlrLe_write/1
  Ground_22/1 -> OvrdForMaxReqForActrForRowFirstAtFlr_OvrdForMaxReqForActrForRowFirstAtFlrRi_write/1
  Ground_23/1 -> OvrdForMaxReqForActrForRowFirstAtVent_OvrdForMaxReqForActrForRowFirstAtVent_write/1
  Ground_24/1 -> OvrdForMaxReqForActrForRowFirstAtVent_OvrdForMaxReqForActrForRowFirstAtVentLe_write/1
  Ground_25/1 -> OvrdForMaxReqForActrForRowFirstAtVent_OvrdForMaxReqForActrForRowFirstAtVentRi_write/1
  Ground_26/1 -> OvrdForMaxReqForActrForRowFirstAtVent_OvrdForMaxReqForActrForRowFirstAtVentSideLe_write/1
  Ground_27/1 -> OvrdForMaxReqForActrForRowFirstAtVent_OvrdForMaxReqForActrForRowFirstAtVentSideRi_write/1
  Ground_28/1 -> OvrdForMaxReqForActrForRowSecAtFlr_OvrdForMaxReqForActrForRowSecAtFlr_write/1
  Ground_29/1 -> OvrdForMaxReqForActrForRowSecAtFlr_OvrdForMaxReqForActrForRowSecAtFlrLe_write/1
  Ground_30/1 -> OvrdForMaxReqForActrForRowSecAtFlr_OvrdForMaxReqForActrForRowSecAtFlrRi_write/1
  Ground_31/1 -> OvrdForMaxReqForActrForRowSecAtVent_OvrdForMaxReqForActrForRowSecAtVent_write/1
  Ground_32/1 -> OvrdForMaxReqForActrForRowSecAtVent_OvrdForMaxReqForActrForRowSecAtVentLe_write/1
  Ground_33/1 -> OvrdForMaxReqForActrForRowSecAtVent_OvrdForMaxReqForActrForRowSecAtVentRi_write/1
  Ground_34/1 -> ResrvdSigForECM4_ResrvdSigForECM4_write/1
